simulink model "MCVT_Integration"  (R2020a)
settings: Solver=ode8  StartTime=0.0  StopTime=T_end  FixedStep=dt  RelTol=1e-3  EnableMultiTasking=on
values from neighbouring files (symbols this model uses):
  assumed_power_voltage = 1.25e2    (= 125)    [MCVT_Input_File.m]    (also in MCVT_Run_QuickCheck.m)
  battery_power_toggle = 1    [MCVT_Input_File.m]    (also in MCVT_Run_QuickCheck.m)
  battery_trickle_charge_param = 0.1    [MCVT_Input_File.m]    (also in MCVT_Run_QuickCheck.m)
  battery_trickle_leakage_param = 0.01    [MCVT_Input_File.m]    (also in MCVT_Run_QuickCheck.m)
  damage_case_id = 3    [MCVT_Input_File.m]    (also in MCVT_Run_QuickCheck.m = 1)
  dt = 1/fs    (= 0.001)    [MCVT_Run_QuickCheck.m]    (also in MCVT_Sim_Set.m)
  dt_2 = 1/fs_2    (= 0.001)    [MCVT_Run_QuickCheck.m]    (also in MCVT_Sim_Set.m)
  dt_3 = 1/fs_3    (= 0.001)    [MCVT_Run_QuickCheck.m]    (also in MCVT_Sim_Set.m)
  dt_5 = 1/fs_5    (= 0.005)    [MCVT_Run_QuickCheck.m]    (also in MCVT_Sim_Set.m)
  dt_8 = 1/fs_8    (= 0.05)    [MCVT_Run_QuickCheck.m]    (also in MCVT_Sim_Set.m)
  dt_9 = 1/fs_9    (= 0.001)    [MCVT_Run_QuickCheck.m]    (also in MCVT_Sim_Set.m)
  dust_met_duration = 3    [MCVT_Input_File.m]    (also in MCVT_Run_QuickCheck.m = 2)
  ECLSS_indoor_set_temperature = 298.15    [MCVT_Input_File.m]    (also in MCVT_Run_QuickCheck.m)
  energy_store_max_o = 150    [MCVT_Input_File.m]    (also in MCVT_Run_QuickCheck.m)
  energy_store_o = 120    [MCVT_Input_File.m]    (also in MCVT_Run_QuickCheck.m)
  fs = 1000    [MCVT_Run_QuickCheck.m]    (also in MCVT_Sim_Set.m)
  gain_charge_param = 1    [MCVT_Input_File.m]    (also in MCVT_Run_QuickCheck.m)
  ground_temperature = 373    [MCVT_Habitat_Design_Input_File.m]    (also in MCVT_Run_QuickCheck.m)
  I_max_charge = 800    [MCVT_Input_File.m]    (also in MCVT_Run_QuickCheck.m)
  I_max_discharge = 800    [MCVT_Input_File.m]    (also in MCVT_Run_QuickCheck.m)
  initial_pressure = 1e5    (= 100000)    [MCVT_Input_File.m]    (also in MCVT_Run_QuickCheck.m)
  initial_temperature = 295    [MCVT_Input_File.m]    (also in MCVT_Run_QuickCheck.m)
  interior_leak_rate = 0.00415    [MCVT_Input_File.m]    (also in MCVT_Run_QuickCheck.m)
  launch_dust_accum_start_time = 10    [MCVT_Input_File.m]    (also in MCVT_Run_QuickCheck.m = 2)
  launch_dust_accum_stop_time = 12    [MCVT_Input_File.m]    (also in MCVT_Run_QuickCheck.m = 3)
  meteorite_impact_time = 2    [MCVT_Input_File.m]    (also in MCVT_Run_QuickCheck.m = 1)
  nuclear_power_toggle = 1    [MCVT_Input_File.m]    (also in MCVT_Run_QuickCheck.m)
  print_interval = 30    [MCVT_Input_File.m]
  SOC_lower_thres = 0.5    [MCVT_Input_File.m]    (also in MCVT_Run_QuickCheck.m)
  SOC_upper_thres = 0.9    [MCVT_Input_File.m]    (also in MCVT_Run_QuickCheck.m)
  solar_power_toggle = 1    [MCVT_Input_File.m]    (also in MCVT_Run_QuickCheck.m)
  space_temperature = 4    [MCVT_Habitat_Design_Input_File.m]    (also in MCVT_Run_QuickCheck.m)
  Start_angle = 90    [MCVT_Input_File.m]    (also in MCVT_Run_QuickCheck.m)
undefined symbols (not defined in the file or in its folder): A_flow_restrictor, ACTIVITY_LOOKUP_TABLE, air, Aref, Bmap, Bmap2, Bmap3, comms, DiscIntNeverNeededParam, DmgSPL, dust_parameters, ECLSS_dust_damage_repair_time, ECLSS_heater_capacity, ECLSS_IPS_restrictor, ECLSS_IPS_setpointPressure, ECLSS_paint_damage_repair_time, ECLSS_radiatorLoop_discreteNumber, ECLSS_Vst, emptyActivity, expected_heat_add, FI, habitat, HIEM2SMM, HIEM2STM, HIEMDamageElements, impact_numbering, initial_SPLT_damage_index, input_air, input_Cond, input_CP, input_discretization, input_EV, input_Evap, input_radiatorLoop, K_ECLSS_dust_single, K_ECLSS_paint_single, K_Structure_damage_single, Kref, kW_to_W, meteoroidDustDep, mFRF, ndelays, nominalDustDep, noverlap, nuclear_toggle_gain, output_limits, panel_paint0, Ps0, reference_temperature, RepairTime_ECLSS_factor, Sensor_Location_SMM, SMM_T0, SMM_T1, SMM_T2, SMM_T3, SMM_T4, SMM_T4_0, SMM_T4_1, SMM_T4_2, solar_FDD_K ... (+73)
libraries: neural x350, simulink x39, xpcutilitieslib x7, xpcrtudplib x7, dspstat3 x1
(part 43 of 43)

== matlab function MCVT_Integration/Sys5 - ECLSS/Interior Pressure Control System (IPCS)/Restrictor ==
  data: Ps input; Afr input; ms output; Pint input; Vop input; ECLSS_IPS_restrictor parameter; air parameter
function ms = flow_restrictor(Ps, Afr, Pint, Vop,  ECLSS_IPS_restrictor, air)
%% Documentation
% Purpose: find mass flow rate of air supply
% Date Created: 20 June 2020
% Date Last Modified: 27 July 2021
% Modeler Name: Jaewon Park, CJ Pan (Purdue)
% Funding Acknowledgement: Funded by the NASA RETHi Project (80NSSC19K1076)

% Version Number: MCVT v1.4

% Subsystem Connections: recieves an input from subsystem 8 and sends an output to subsystem 8

%%% All inputs and outputs to this function are 1 dimensional %%%
% INPUTS %
%   - Ps: air storage tank pressure, unit: Pa, type: float
%   - Pint: interior environment pressure, unit: Pa, type: float
%   - Afr: effective cross-sectional area of flow restrictor, unit: m^2, type: float
%   - Vop: valve opening factor, used for damageability case (TO DO)
%   - ECLSS_IPS_restrictor: flow restrictor design parameters, type: struct (see below for dot indices)
%                           permeability: Permeability of the flow restrictor, unit: m^2, type: float
%                           length: Length of the flow restrictor, unit:m, type: float
%                           channel number: number of small channels in restrictor, unit: none, type: float
%                           cross area: Flow restrictor area (cross-section), unit: m^2, type: float
%   - air: air material property, type: struct (see below for dot indices)
%                           specific heat: Specific heat of air, unit: J/(kg.k), type: float
%                           density: density of air, unit: kg/m^3, type: float
%                           viscosity: dynamic viscosity of air, unit: Pa*s, type: float
    
% OUTPUTS %
%   - ms: mass flow rate of air supply, unit: kg/s, type: float

% Function Dependencies: No dependency

% No Data Depenencies

%% Calculate mass flow rate through a flow restrictor
ms=ECLSS_IPS_restrictor.channel_number * Vop * Afr * air.density *...
    ECLSS_IPS_restrictor.permeability / air.viscosity * (Ps-Pint) / ...
    ECLSS_IPS_restrictor.length;                                        % mass flow rate of supplied air


end

== matlab function MCVT_Integration/Sys5 - ECLSS/Active Thermal Control System (ATCS)/tempadjust ==
  data: x input; y output; Tset input; t input
function y= tempadjust(x, Tset, t)
%% Documentation
% Purpose: Filter out temperature readings that are not a number 
% Date Created: 20 June 2020
% Date Last Modified: 1 Sept 2021
% Modeler Name: Yuguang Fu(Purdue)
% Funding Acknowledgement: Funded by the NASA RETHi Project (80NSSC19K1076)

% Version Number: MCVT v1.4

% Subsystem Connections: receives input from subsystem 8

%%% All inputs and outputs to this function are 1 dimensional %%%
% INPUTS %
%   - x: temperature reading from interior habitat, unit: C, type: float
%   - Tset: setpoint temperature, unit: C, type: float
%   - t: current simulation time, unit: s, type: float
    
% OUTPUTS %
%   - y: filtered temperature, unit: C, type: float

% Function Dependencies: No dependency

% No Data Depenencies
%% Filtering
    if isnan(x) && t<=0.105 % if the temperature reading is not a number and it is early stage of the simulation  
        %fprintf('Interior ENV temperature as nan')
        y=Tset;             % assume the reading is the same as temperature setpoint 
    elseif isnan(x)
        y=Tset;             % assume the reading is the same as temperature setpoint 
    else                    
        y=x;                % otherwise, just read the value as it is 
    end

    
% This is happening because at each subsystem is run at different
% frequencies. Interior habitat has the lowest frequency. Therefore, it
% does not output any value during the beginning stage of the simulation. 
% This problem needs to be solved (not by ECLSS obvisouly). This is only for temporary fix. 
end

== matlab function MCVT_Integration/Sys2 - Structural System/Sys2_Structural_System/Structural Mechanical 2.1/Cat Acceleration ==
  data: Acc input; Dom input; Acc_Sen output; SMM_T4_0 parameter; Sensor_Location_SMM parameter
function Acc_Sen = Cat_acceleration(Acc,Dom, SMM_T4_0,Sensor_Location_SMM)

%% This block extract acceleration for the given sensor location


%% SL contains sensor locations
% Select the closest nodes to given sensor locations
% Safe: Can work with any input coordinate
    [SN]=Sensor_Locator(Sensor_Location_SMM,SMM_T4_0.Nodes_M(:,:,Dom));
    
%% Get the acceleration only to the Sensors    
    Acc_Sen=zeros(length(SN)*3,1);
    for i=1:length(SN)
        
        Acc_Sen(3*i-2:3*i,1)=Acc(3*SN(i)-2:3*SN(i),1);
        
    end


end


function[SN,b]=Sensor_Locator(SL,Nodes)
   
    S=size(Nodes);
    nnp1=S(1);

    S=size(SL);
    SN=zeros(S(1),1);
    for i=1:S(1)
        
        D=zeros(nnp1,1);
        for k=1:nnp1
            D(k)=norm([Nodes(k,2) Nodes(k,3),Nodes(k,4)]-[SL(i,1),SL(i,2),SL(i,3)]);
        end
        
        [a,b]=min(D);
        
        SN(i)=Nodes(b,1);
    end 
        
end

== matlab function MCVT_Integration/Sys2 - Structural System/Sys2_Structural_System/Structural Mechanical 2.1/Domain Selection ==
  data: ILxyz input; Dom output; T_Node output; SMM_T4_0 parameter; T_NodeI input; DomI input; t input
function [Dom,T_Node] = Domain_Selection(ILxyz,SMM_T4_0,T_NodeI,DomI,t)
%% This block sets the Domain based on Impact location
% Inputs: 
%       ILxyz: Impact coordinate 
%       SMM_T4_0.P_N_M: Set of permissible Impact nodes on SMM
%       SMM_T4_0.Nodes_M: Matrix containing Node set. SMM_T4_0.Nodes_M(:,:,1) represents Nodes when Domain 1 forms Kbb matrix
%       T_NodeI: Target node input
%       DomI: Domain no input
%       t: Time

% Outputs:
%       Dom: Domain no output
%       T_Node: Target node output

%% Set previous as new to avoid Domain selection computation at each step

   T_Node=T_NodeI;  % Output Target Node set to Input Target Node (Impact node)
   Dom=DomI;        % Output Domain set to Input Domain

%% Select and Fix the domain only at the beginning of simulation
if t==0

    % This function searh for the closest Node and element 
        % Safe: Can work with any Impact location!
        [~,b]=Impact_Locator(ILxyz,SMM_T4_0.Nodes_M(SMM_T4_0.P_N_M(:,:,1),:,1));

    % Set the domain
        Dom=b;

    % Set the target node
        T_Node=SMM_T4_0.P_N_M(1,b,b);

end
    
    

end

%% Find closest node number SN and node array location b
function[SN,b]=Impact_Locator(SL,Nodes)

    % Input given node: SL format: [Node 1, Node 2,... Node i,... Node end]

    S=size(Nodes);
    nnp1=S(1);

    % If given SL Coordinates are in wrong format, make it right by transposing
    S=size(SL);
    if S(2)>1
        SL1=SL';
    else
        SL1=SL;
    end
    
    % Get the distance vector for each node
        D=zeros(nnp1,1);
        for k=1:nnp1
            D(k)=norm([Nodes(k,2) Nodes(k,3),Nodes(k,4)]-[SL1(1,1),SL1(2,1),SL1(3,1)]);
        end

    % Find the minimum
        [a,b]=min(D);

    % Set the Node number      
        SN=Nodes(b,1);

end

== matlab function MCVT_Integration/Sys2 - Structural System/Sys2_Structural_System/Structural Thermal Model 2.2/STM Temperature Sensors/ Resistance Temperature Detector1/T vs R ==
  data: R output; T input
function R = fcn(T)

if T >= 0
    R = 100*(1+3.9083*10^(-3)*T-5.775*10^(-7)*T^2);
else
    R = 100*(1+3.9083*10^(-3)*T-5.775*10^(-7)*T^2-4.183*10^(-12)*(T-100)*T^3);
end

== matlab function MCVT_Integration/Sys2 - Structural System/Sys2_Structural_System/Structural Thermal Model 2.2/STM Temperature Sensors/ Resistance Temperature Detector2/Power Consumption ==
  data: Supply input; kWUsage3 output; Switch input
function kWUsage3 = Power_Consumption(Supply,Switch)

if Switch >= 1
    Voltage = 5.5; %Volts
    Current = 0.000001; %10 microAmperes
    
    kWUsage3 = (Voltage * Current)/1000;
    
    if kWUsage3 >= Supply
        kWUsage3 = Supply;
    end
    
else
    kWUsage3 = 0;
end
end

== matlab function MCVT_Integration/Sys2 - Structural System/Sys2_Structural_System/Structural Thermal Model 2.2/STM Temperature Sensors/ Resistance Temperature Detector2/R vs T ==
  data: R input; T output
function T = fcn(R)

T = 1.76520388802152e-09 * R^4 - 2.98990866289637e-07 * R^3 + 0.000987249006463873 * R^2 ...
    + 2.36178487086887 * R - 245.924688605077;

end

== matlab function MCVT_Integration/Sys2 - Structural System/Sys2_Structural_System/Structural Thermal Model 2.2/STM Temperature Sensors/ Resistance Temperature Detector2/RTD with Self-heat Error/MATLAB Function ==
  data: I input; R input; U output
function U = fcn(I, R)

U = I * R;     % Operating current = 1mA
               % Voltage output U = I*R

== matlab function MCVT_Integration/Sys2 - Structural System/Sys2_Structural_System/Structural Thermal Model 2.2/STM Temperature Sensors/ Resistance Temperature Detector2/RTD without Self-heat Error/MATLAB Function ==
  data: I input; I output; R input; U output
function [I,U]= fcn(I,R)

U = I * R;     % Operating current = 1mA
               % Voltage output U = I*R

== matlab function MCVT_Integration/Sys2 - Structural System/Sys2_Structural_System/Structural Thermal Model 2.2/STM Temperature Sensors/ Resistance Temperature Detector2/Self-heat Calculation/  T vs R  ==
  data: R output; T input
function R = fcn(T)

if T >= 0
    R = 100*(1+3.9083*10^(-3)*T-5.775*10^(-7)*T^2);
else
    R = 100*(1+3.9083*10^(-3)*T-5.775*10^(-7)*T^2-4.183*10^(-12)*(T-100)*T^3);
end

== matlab function MCVT_Integration/Sys2 - Structural System/Sys2_Structural_System/Structural Thermal Model 2.2/STM Temperature Sensors/ Resistance Temperature Detector2/Switch ON//OFF Control Block/Switch OFF/MATLAB Function1 ==
  data: u input; y output; Time input
function y = fcn(u,Time)

if Time>=0
    u = NaN;
end

y = u;

== matlab function MCVT_Integration/Sys2 - Structural System/Sys2_Structural_System/Structural Thermal Model 2.2/STM Temperature Sensors/ Resistance Temperature Detector2/T vs R ==
  data: R output; T input
function R = fcn(T)

if T >= 0
    R = 100*(1+3.9083*10^(-3)*T-5.775*10^(-7)*T^2);
else
    R = 100*(1+3.9083*10^(-3)*T-5.775*10^(-7)*T^2-4.183*10^(-12)*(T-100)*T^3);
end

== matlab function MCVT_Integration/Sys2 - Structural System/Sys2_Structural_System/Structural Thermal Model 2.2/STM Temperature Sensors/ Switch ON//OFF Control Number4 ==
  data: Power input; Switch output
function Switch = Switch2(Power)

if Power <= 5.5e-9
    Switch = 2;
else
    Switch = 1;
end

end

== matlab function MCVT_Integration/Sys2 - Structural System/Sys2_Structural_System/Structural Thermal Model 2.2/STM Temperature Sensors/Cumulative_Power_STM ==
  data: TP input; CTP output; PrevCTP input
function CTP = Cumulative_Power_STM(TP,PrevCTP)

CTP = TP+PrevCTP;

end

== matlab function MCVT_Integration/Sys2 - Structural System/Sys2_Structural_System/Structural Thermal Model 2.2/STM Temperature Sensors/Sensor Input to CDCM ==
  data: RightBottom input; Wall_Temp_Sensed output; Zenith input; LeftBottom input
function Wall_Temp_Sensed = Sensor_Placement(RightBottom,Zenith,LeftBottom)

a = RightBottom(1,1);
b = Zenith(1,1);
c = LeftBottom(1,1);

Wall_Temp_Sensed = ones(3,1);

Wall_Temp_Sensed(1,1) = a;
Wall_Temp_Sensed(2,1) = b;
Wall_Temp_Sensed(3,1) = c;

end

== matlab function MCVT_Integration/Sys2 - Structural System/Sys2_Structural_System/Structural Thermal Model 2.2/STM Temperature Sensors/Sensor Placement ==
  data: Wall_Temp input; RightBottom output; Zenith output; LeftBottom output
function [RightBottom,Zenith,LeftBottom] = Sensor_Placement(Wall_Temp)

Locations = [1 5 11];

Temp_to_Sensor = Wall_Temp(Locations,1);

RightBottom = Temp_to_Sensor(1,1);
Zenith = Temp_to_Sensor(2,1);
LeftBottom = Temp_to_Sensor(3,1);

end

== matlab function MCVT_Integration/Sys 6 - Health Management and Agents/Decision Maker/Simulink Communication Modules/ECLSS_Dust//MCVT API to HMS/UDP_Send/MATLAB Function1 ==
  data: SizeInfo output; signal input
function SizeInfo=size_Info(signal)

info=size(signal);

SizeInfo=[info(1), info(2)];

== matlab function MCVT_Integration/Sys 6 - Health Management and Agents/Decision Maker/Simulink Communication Modules/ECLSS_Dust//MCVT API to HMS/module2 ==
  data: u_t input; u_av output; u_std output; buffSize input
function [u_av, u_std] = buffer_average(u_t, buffSize)

    if buffSize < 10
        buffSize = 10;
    end
    % initialize buffer
    [nR, ~] = size(u_t);
    
    persistent count;
    persistent u_arr;
    
    if isempty(count)
        count = 0;
        u_arr = zeros(buffSize,nR);
    end
    
    % shuffle the elements down
    u_arr(2:end,1:end) = u_arr(1:end-1,1:end);
    
    % add the new element
    u_arr(1,1:end) = u_t;
    
    % average
    if count == 0
        u_av = u_t;
        u_std = zeros(size(u_t));
        count = count + 1;
    elseif count < buffSize
        u_av = mean(u_arr(1:count+1, 1:end), 1)';
        u_std = std(u_arr(1:count+1, 1:end), 0, 1)';
        count = count + 1;
    else
        u_av = mean(u_arr, 1)';
        u_std = std(u_arr, 0, 1)';
        count = count + 1;
    end
        
end

== matlab function MCVT_Integration/Sys 6 - Health Management and Agents/Decision Maker/Simulink Communication Modules/ECLSS_Paint//MCVT API to HMS/UDP_Send/MATLAB Function ==
  data: hi0 output; len input; hdr_Info input; rowcol input; len0 output
function [hi0, len0]=headerInfo(len, hdr_Info, rowcol)
src=hdr_Info(1);
dest=hdr_Info(2);
type=hdr_Info(3);
prio=hdr_Info(4);
row=rowcol(1); 
col=rowcol(2);
% len=0; 
hi0=[src, dest, type, prio, row, col];
len0=len;
% hi=uint8(hi);

== matlab function MCVT_Integration/Sys 6 - Health Management and Agents/Decision Maker/Simulink Communication Modules/ECLSS_Paint//MCVT API to HMS/UDP_Send/MATLAB Function1 ==
  data: SizeInfo output; signal input
function SizeInfo=size_Info(signal)

info=size(signal);

SizeInfo=[info(1), info(2)];

== matlab function MCVT_Integration/Sys 6 - Health Management and Agents/Decision Maker/Simulink Communication Modules/ECLSS_Paint//MCVT API to HMS/module2 ==
  data: u_t input; u_av output; u_std output; buffSize input
function [u_av, u_std] = buffer_average(u_t, buffSize)

    if buffSize < 10
        buffSize = 10;
    end
    % initialize buffer
    [nR, ~] = size(u_t);
    
    persistent count;
    persistent u_arr;
    
    if isempty(count)
        count = 0;
        u_arr = zeros(buffSize,nR);
    end
    
    % shuffle the elements down
    u_arr(2:end,1:end) = u_arr(1:end-1,1:end);
    
    % add the new element
    u_arr(1,1:end) = u_t;
    
    % average
    if count == 0
        u_av = u_t;
        u_std = zeros(size(u_t));
        count = count + 1;
    elseif count < buffSize
        u_av = mean(u_arr(1:count+1, 1:end), 1)';
        u_std = std(u_arr(1:count+1, 1:end), 0, 1)';
        count = count + 1;
    else
        u_av = mean(u_arr, 1)';
        u_std = std(u_arr, 0, 1)';
        count = count + 1;
    end
        
end

== matlab function MCVT_Integration/Sys 6 - Health Management and Agents/Decision Maker/Simulink Communication Modules/NPG_Dust//MCVT API to HMS/Buffer Average ==
  data: u_t input; u_av output; u_std output; buffSize input
function [u_av, u_std] = buffer_average(u_t, buffSize)
    

    if buffSize < 10
        buffSize = 10;
    end
    % initialize buffer
    [nR, ~] = size(u_t);
    
    persistent count;
    persistent u_arr;
    
    if isempty(count)
        count = 0;
        u_arr = zeros(buffSize,nR);
    end
    
    % shuffle the elements down
    u_arr(2:end,1:end) = u_arr(1:end-1,1:end);
    
    % add the new element
    u_arr(1,1:end) = u_t;
    
    % average
    if count == 0
        u_av = u_t;
        u_std = zeros(size(u_t));
        count = count + 1;
    elseif count < buffSize
        u_av = mean(u_arr(1:count+1, 1:end), 1)';
        u_std = std(u_arr(1:count+1, 1:end), 0, 1)';
        count = count + 1;
    else
        u_av = mean(u_arr, 1)';
        u_std = std(u_arr, 0, 1)';
        count = count + 1;
    end
        
end

== matlab function MCVT_Integration/Sys2 - Structural System/Sys2_Structural_System/Structural Mechanical 2.1/Solver ==
  data: Disp1 output; Disp0 input; Acc0 input; Vel0 input; Kbar input; D_Eq input; Vel1 output; Acc1 output; KbarO output; D_EqO output; Ext_F input; In_Acc input; Fadd input; Repair_Status input; FaddO output; Damage_Status input; Damage_Status_O output; Dom input; Im_F input; t input; Total_Damage output; SMM_T0 parameter; SMM_T1 parameter; SMM_T4_1 parameter; SMM_T2 parameter; SMM_T3 parameter; SMM_T4 parameter
function [Disp1,Vel1,Acc1,KbarO,D_EqO,FaddO,Damage_Status_O,Total_Damage]=Solver_Scheme_Sim(Disp0,Acc0,Vel0,Kbar,D_Eq,Ext_F,In_Acc,Fadd,Repair_Status, Damage_Status, Dom,Im_F,t,SMM_T0, SMM_T1, SMM_T4_1, SMM_T2, SMM_T3, SMM_T4)


%% This block Solves 3D Structural dynamics Equation: Md^2u/dt^2+Cdu/dt+Ku=F and calculate Damage and Repair
% Inputs ( not from Main\Workspace):
%       Ext_F: External Force vector
%       In_Acc: External acceleration
%       Im_F: Impact force
%       Fadd: Additional Force vector to stabilize excitation during repair at previous timestep
%       Repair_Status: Repair data from Agent model
%       Dom: Domain no
%       t: Time
%       Kbar: Mass normalized Stiffness matrix at previous timestep
%       D_Eq: Damage matrix at previous timestep
%       Damage_Status: List format of Damage matrix
%       Disp0: Displacement at previous timestep
%       Vel0: Velocity at previous timestep
%       Acc0: Acceleration at previous timestep

% Inputs from Main\Workspace
%       SMM_T0, SMM_T1, SMM_T4_1, SMM_T2, SMM_T3, SMM_T4: Read SysFiles\Subs2\SMM_Readme.txt

% Outputs: 
%       Disp1: Displacement at current timestep
%       Vel1: Velocity at current timestep
%       Acc1: Acceleration at current timestep
%       FaddO: Additional Force vector to stabilize excitation during repair at current timestep
%       KbarO: Mass normalized Stiffness matrix at current timestep
%       D_EqO: Damage matrix at current timestep
%       Damage_StatusO: List format of Damage matrix at current timestep



%% Damp external force at the beginning of simulation
    % Purpose:
        % At t=0 there is no force. At t=dt, Thermal+Internal Pressure+Gravity acts on the model
        % This sudden force cause unwanted excitation to the model.
        % This section multiply a corrective term CorrM to damp the force where force gradually increases for 1 sec instead of dt sec.
    
    if t<=1 %1 sec
        % Fourth order damping fit constants
            p1 =       1.182;
            p2 =        -2.2;
            p3 =     -0.1348;
            p4 =       2.153;
            p5 =   3.385e-16;
        % Corrective term to multiply with external force
            CorrM= p1*t^4+p2*t^3+p3*t^2+p4*t +p5;

        % Set maximum multiplyer to 1
            if CorrM>1
               CorrM=1;
            end
        
        % Set the damped external force vector
            Ext_F=Ext_F*CorrM;   
    end


%% Equivalent nodal force from Acceleration calculation
    % Summary:
        % Force=Mass*Acceleration. 
        % SMM_T4.M_M(:,:,Dom): Mass matrix associated to Domain Dom
        % In_Acc(1:SMM_T3.L,1): Chop the acceleration to L dergrees of freedom
    
    ext_A=SMM_T4.M_M(:,:,Dom)*In_Acc(1:SMM_T3.L,1);
                        
%% The Newmark method (constant acceleration)    
    % Purpose:
        % This section solves Md^2u/dt^2+Cdu/dt+Ku=F 
        % For details look into the Documentation
        % Equivalent terms for this code
            % M             : SMM_T4.M_M(:,:,Dom)
            % C             : SMM_T4.C_M(:,:,Dom)
            % K             : SMM_T4.K_M(:,:,Dom)
            % Kbar          : Kbar(:,:,Dom)
            % (t+delt)^F    : Ext_F(1:SMM_T3.L,1)+ext_A
            % a0-a7         : SMM_T1.a0-SMM_T1.a7
            

    % Equivalent Force vector calculation
        Rbar=SMM_T4.M_M(:,:,Dom)*(SMM_T1.a0*Disp0+SMM_T1.a2*Vel0+SMM_T1.a3*Acc0)+SMM_T4.C_M(:,:,Dom)*(SMM_T1.a1*Disp0+SMM_T1.a4*Vel0+SMM_T1.a5*Acc0)+Fadd(1:SMM_T3.L,1)+(Ext_F(1:SMM_T3.L,1)+ext_A);  
          
        fi=Rbar(1:SMM_T4.Li,1);
        fb=Rbar(SMM_T4.Li+1:end);

    % Calculation for domain decomposition
        Kbb=Kbar(SMM_T4.Li+1:end,SMM_T4.Li+1:end,Dom);
        Rb=chol(Kbb-SMM_T4.Kbbr_M(:,:,Dom));
        f_eq_b=fb-SMM_T4.fbr_M(:,:,Dom)*fi;
        
    % Displacement vectors for each domain 
        db = Rb\(Rb'\f_eq_b);                                       
        di = SMM_T4.IKii_M(:,:,Dom)*(fi-SMM_T4.Kib_M(:,:,Dom)*db);

    % Total displacement vector
        Disp1=[di;db];    

    % Solve for the corresponding velocities and accelerations
        Acc1=SMM_T1.a0*(Disp1-Disp0)-SMM_T1.a2*Vel0-SMM_T1.a3*Acc0;
        Vel1=Vel0+SMM_T1.a7*Acc1+SMM_T1.a6*Acc0; 
        
            
%% Damage Calculation 
    % Purpose:
        % This section reduce stiffness from the impacted element to replicate Damage
        
        
    % Get the element that is impacted
        e=SMM_T0.Reg_Elems(Dom);   

    % ---------------Get damage from data driven model------------
    % Hard coded for efficiency
    % This data driven model has been developed around March 2021

    % Damage constant
        Im_F=abs(Im_F)/10^6;            % Impact force in MPA
        dam_26=48.52*Im_F^0.1331-40;    % Damage constant near outer surface
        dam_27=48.52*Im_F^0.1331-40-8;  % Damage constant at the middle of element
        dam_21=48.52*Im_F^0.1331-40-10; % Damage constant near inner surface

    % Damage volume (m^3)
        Vl=zeros(3,1);
        if dam_26>0
            Vl(1)=dam_26*0.133333^3*10; % Damage volume near outer surface
        end
        if dam_27>0
            Vl(2)=dam_27*0.133333^3*6;  % Damage volume at the middle of element
        end
        if dam_21>0
            Vl(3)=dam_21*0.133333^3*4.5;% Damage volume near inner surface
        end

    %------------Remove Stiffness from Element--------- 
    % Safe: Work with any Input model. Doesn't crash
    c=1;
    for gp=[26 27 21]   % 26, 27 and 21 Gauss points are at the center of element

        if sum(Vl)>0  && D_Eq(gp,e)==0% Switch calculation if Volume loss detected

            % Get total stiffness for the element e
                KeT=SMM_T3.Ke(1:SMM_T0.Type(2,e)*3,1:SMM_T0.Type(2,e)*3,gp,e);

            % Determine the Damage (Stiffness loss ratio) from Volume loss    
                Mag=(Vl(c)/(SMM_T2.dJB(gp,e)*SMM_T1.W(gp,SMM_T0.Type(1,e))));

            % Stop damage from exceeding 1.5
                if Mag>1.5
                    Mag=1.5;
                    fprintf('        Warning! Damage is too high! Restricted to 1.5\n');
                end

            % Updated stiffness matrix    
                KeU=KeT*(1-Mag);

            % Remove Gauss point Stiffness from Global stiffness
                for loop1 = 1:SMM_T0.Type(2,e)*3
                    k = SMM_T4_1.LM_M(loop1,e,Dom);
                    for loop2 =  1:SMM_T0.Type(2,e)*3
                       j = SMM_T4_1.LM_M(loop2,e,Dom);
                       if k<=SMM_T3.L && j<=SMM_T3.L % Exclude D.O.F. associated with fixed end Boundary
                           % New Kbar    = Previous Kbar+Updated K       -Total K    
                           Kbar(k,j,Dom) = Kbar(k,j,Dom)+KeU(loop1,loop2)-KeT(loop1,loop2);     
                        end
                    end
                end

            % Update damage to D_Eq
                D_Eq(gp,e)=Mag;

        end 
        c=c+1; % Keep track of V1 vector
    end            
            
            
%% Repair Calculation    
    % Purpose:
        % This section Add stiffness to the damaged element to replicate Repair
        % This also determines additional force vector Fadd to exclude excitation to to repair
        
    % Set repair rate
        Repair_Rate= Repair_Status(4,1);    % Get repair rate (m^3)/0.001sec

    % Distribute repair rate to damaged Gauss points
        S=length(find(D_Eq>10^-3));
        if S>0
            RateI=Repair_Rate/S; % Distribute repair amount among Damaged gauss points
        else
            RateI=Repair_Rate;   % When no Damaged gauss points are there the code still runs w/o error
        end

    %------------------Add Stiffness to Element due to repair--------- 
    % Safe: Work with any Input model. Repair never goes over healthy state.   
        if Repair_Status(1,1)>10^-3 % Switch on repair action when Agent is enganged. Repair_Status(1,1)=1 

            for gp=1:SMM_T0.Type(3,e)   % Runs through all the Gauss points

                if D_Eq(gp,e)>10^-5  % Switch on repair action further if Damage amount >Tolerance

                    % Determine the Repair (Stiffness Gain ratio) from Volume gain
                        Mag_rep=RateI/(SMM_T2.dJB(gp,e)*SMM_T1.W(gp,SMM_T0.Type(1,e))); 

                    % If Repaired amount exceeds healty amount adjust Repair 
                        RP=0;
                        if Mag_rep>D_Eq(gp,e)
                            Mag_rep=D_Eq(gp,e); % Set repair to make Damage=0
                            RP=1;
                        end

                    % Previous 1-Damage
                        MagP=1-D_Eq(gp,e);

                    % New 1-Damage
                        MagN=1-(D_Eq(gp,e)-Mag_rep);

                        if MagN>1
                            MagN=1;
                        end
                        
                    % Get total stiffness for the element e
                        KeT=SMM_T3.Ke(1:SMM_T0.Type(2,e)*3,1:SMM_T0.Type(2,e)*3,gp,e);

                    % Get Stiffness to be added to Kbar
                        dKe=(MagN-MagP)*KeT;

                    % Add Gauss point Stiffness to Global stiffness 
                        for loop1 = 1:SMM_T0.Type(2,e)*3
                            k = SMM_T4_1.LM_M(loop1,e,Dom);
                            for loop2 =  1:SMM_T0.Type(2,e)*3
                               j = SMM_T4_1.LM_M(loop2,e,Dom);
                               if k<=SMM_T3.L && j<=SMM_T3.L % Exclude Boundaru D.O.F.
                                   % New Kbar    = Old Kbar     +Added Kbar
                                   Kbar(k,j,Dom) = Kbar(k,j,Dom)+dKe(loop1,loop2);
                                end
                            end
                        end

                    % Set the Damage matrix D_Eq
                        if RP==1
                            D_Eq(gp,e)=0;
                        else
                            D_Eq(gp,e)=(D_Eq(gp,e)-Mag_rep);
                        end

                end
            end

            % Add Correction Force vector to damp acceleration 
                Fadd(1:SMM_T3.L)=Fadd(1:SMM_T3.L)+Kbar(:,:,Dom)*Disp1-Rbar; 


        end


%% Set damage output for other models
    % Purpose:
        % Convert Damage matrix D_Eq (Which is required for SMM computation) to a Vector: Damage_Status, which can be used by other subsystems
        
    i=1;
    % SM.Damage_Status=SM.Damage_Status*0;
    for e=1:SMM_T1.nel%1:SM.SMM_T1.nel%[1 2 9 10 17 18]%1:SM.SMM_T1.nel % CPU parallelization may be done, making multiple domains of elements                      
           for gp=1:SMM_T0.Type(3,e)  % Complete parallelization may be done with GPU, each GPU processor for each gauss point, the for loop for element may go away

                  Damage_Status(i,1)=[D_Eq(gp,e)];

               i=i+1;
           end
    end
               
                    
    Damage_Status_O=Damage_Status;
    Total_Damage=sum(sum(D_Eq));%sum(Damage_Status);
            
%% Setting output different name then input

    KbarO=Kbar;
    D_EqO=D_Eq;
    FaddO=Fadd;
            
end

== matlab function MCVT_Integration/Sys2 - Structural System/Sys2_Structural_System/Fault Detection and Diagnosis (FDD) with Binary Classifer/Accelerometer/Accelerometer/ Switch ON//OFF Control Number ==
  data: p input; y output
function y = fcn(p)

if p >= 1.2*10^(-4)*3
    u = 1;
else
    u = 2;
end

y = u;

== matlab function MCVT_Integration/Sys2 - Structural System/Sys2_Structural_System/Fault Detection and Diagnosis (FDD) with Binary Classifer/Accelerometer/Accelerometer/Accelerometer/Maximum//minimum Output Voltage ==
  data: u input; y output
function y = fcn(u)

y = u(1:9);

if u(1:9) > 17
    y = 17*ones(9,1);
else
    if u(1:9) < 7
        y = 7*ones(9,1);
    end
end

== matlab function MCVT_Integration/Sys2 - Structural System/Sys2_Structural_System/Fault Detection and Diagnosis (FDD) with Binary Classifer/Accelerometer/Accelerometer/Damage State Control Block ==
  data: state_number input; y output; Acc input; Time input
function y = fcn(state_number,Acc,Time)

for i = 1:size(state_number,1)
    if state_number(i) == 1
        Acc(i*3-2:i*3) = Acc(i*3-2:i*3);
    else
        if state_number(i) == 2
            temp = Acc(i*3-2:i*3) - 12;
            Acc(i*3-2:i*3) = temp * 0.1 + 12;
        else
            if state_number(i) == 3
                if Time>=11 && Time<=14
                    Acc(i*3-2:i*3) = NaN;
                end
            else
                if state_number(i) == 4
                    Acc(i*3-2:i*3) = NaN;
                end
            end
        end
    end
end

y = Acc;

== matlab function MCVT_Integration/Sys2 - Structural System/Sys2_Structural_System/Fault Detection and Diagnosis (FDD) with Binary Classifer/Accelerometer/Accelerometer/Switch ON//OFF Control Block/Switch OFF/MATLAB Function1 ==
  data: sensor input; u input; y output; Time input
function y = fcn(sensor,u,Time)

for i = 1:size(sensor,1)
    if Time>=0
        u(sensor(i)*3-2:sensor(i)*3) = NaN;
    end
end

y = u;

== matlab function MCVT_Integration/Sys2 - Structural System/Sys2_Structural_System/Fault Detection and Diagnosis (FDD) with Binary Classifer/Accelerometer Power Consumption ==
  data: p input; y output
function y = fcn(p)

if p >= 1.2*10^(-4)*3
    u = 1.2*10^(-4)*3;
else
    u = 0;
end

y = u;

== matlab function MCVT_Integration/Sys2 - Structural System/Sys2_Structural_System/Fault Detection and Diagnosis (FDD) with Binary Classifer/Data Anomaly Detection/Data Anomaly Detection ==
  data: Acc input; indicator output; max_value output; num_nan output; y output
function [indicator,max_value,num_nan,y] = fcn(Acc)

indicator = zeros(9,1);
num_nan = zeros(9,1);
max_value = zeros(9,1);

for i = 1:9
    
    y = Acc(:,i);
    TF = isnan(Acc(:,i));
    num_nan(i) = sum(TF);
    max_value(i) = max(abs(Acc(:,i)));
    
    if num_nan(i) == 0
        indicator(i) = 1;
    else
        if num_nan(i) >= 1 && num_nan(i) < 5120
            indicator(i) = 3;
        else
            if num_nan(i) == 5120
                indicator(i) = 4;
            end
        end
    end
    
    if indicator(i) == 1 && max_value(i) <= 3e-3 || max_value(i) >= 1   % modified to 1
        indicator(i) = 2;
    end   
end

== matlab function MCVT_Integration/Sys2 - Structural System/Sys2_Structural_System/Fault Detection and Diagnosis (FDD) with Binary Classifer/FRF from Accelerometer 1/FRF Calculation ==
  data: exc input; frf output; acc input; m input; noverlap input; w input
function frf = xfunc(exc,acc,m,noverlap,w)

%  [XFER21,FREQV,P] = XFUNC(X,Y,FS,M,NOVERLAP) will estimate the
%	complex transfer function given time histories of the
%	input, X, and the output, Y. Given the complex response,
%	the magnitude and phase can be determined by using the
%	ABS and ANGLE functions. FS is the sampling frequency (Hz)
%	at which the data was taken. The X and Y sequences of
%	N points are divided into NS sections of M points each
%	(M must be a power of two). Using an M-point FFT,
%	successive sections are Hanning windowed, FFT'd and
%	averaged.	** SOME PARTS FROM SPECTRUM FUNCTION
%
%	XFUNC(X,Y,FS) 	--> NO AVERAGING
%	XFUNC(X,Y,FS,M)	--> AVERAGING BUT NO OVERLAP
%	NO OUTPUT ARGUMENTS WILL CREATE A BODE PLOT
%
% 	The output P is the matrix of power spectrums in the order
%	P = [Pxx,Pyy,Pxy].
%
%	NOTES: 	Xfer data may be written to a specified file.
%		Variables were chosen to immitate certain IP names.
%		Depending on the anti-aliasing filter used a certain
%			percentage of the data will include aliased
%			data (IP is 78% correct).

% COMMENTS
% nargin = 3 --> no overlap, no averaging
% nargin = 4 --> no overlap, averaging data
% nargin = 5 --> some overlap, and averaging data
% set color variable to easily change plot color
% may also write data to a file

frf = zeros(1,256*3);
x = exc;

for k = 1:1:3
    y = acc(:,k);
    
%     color	= 'b';
%     
%     if (nargin == 3), m = length(y); noverlap = 0; fmax = fs/2; end
%     if (nargin == 4)
%         if (length(y) == 1)
%             noverlap = m;
%             m = length(y);
%         else
%             noverlap = 0;
%         end
%         fmax = fs/2;
%     end
    %keyboard
    x 	= x(:);		% Make sure x and y are column vectors
    y 	= y(:);
    
    % DEFINE CONSTANTS
     n 	= max(size(x));	% Number of data points in entire time history
     ns 	= fix((n-noverlap)/(m-noverlap));	% Number of samples (frames)
    % (k = fix(n/m) for noverlap=0)
%     w 	= hann(m);	% hanning is replaced by hann
    % Type of windowing: change this for application
    % (Try HAMMING, BLACKMAN, BARTLETT, or your own)
    % HANNING is best for narrow-band random !!
     KMU 	= ns*norm(w)^2;	% Norm. scale factor (AVERAGING & WINDOWING)
     index 	= 1:m;
    % fprintf(1,['\n%5i',' Samples Averaged\n'],ns)
    
    
    % FIND POWER SPECTRUMS OF EACH SAMPLE AND SUM
    Pxx = zeros(m,1)+0i; 
%     Pyy = Pxx; 
    Pxy = Pxx; % Plus 0i
    for i = 1:ns
        xw 	= w.*x(index);		% detrend? no difference
        yw 	= w.*y(index);
        index 	= index + (m - noverlap);
        Xx 	= fft(xw);
        Yy 	= fft(yw);
        Pxx 	= Pxx + Xx.*conj(Xx);
%         Pyy 	= Pyy + Yy.*conj(Yy);
        Pxy 	= Pxy + Yy.*conj(Xx);
    end
    
    % SELECT
    %select = [1:m/2];
    select = [1:m/2+1];   % include DC AND Nyquist
    %FreqV	= [fs/2/(m/2):fs/2/(m/2):fs/2]';		% (Nyquist)
%     FreqV	= (select - 1)'*fs/m;
%     mcorrect	= round(.78*max(select));		% first 78%
%     fcorrect	= FreqV(mcorrect);
    
    % AVERAGED & SCALED DATA
    Pxx = Pxx(select)./KMU;			% Double sided
%     Pyy = Pyy(select)./KMU;
    Pxy = Pxy(select)./KMU;
%     P   = [Pxx,Pyy,Pxy];
    
    Xfer21 	= Pxy./Pxx;
    frf(1,256*(k-1)+1:256*k) = abs(Xfer21(1:256,1));
end

% %keyboard
% % NO OUTPUT ARGUMENTS, PLOT BODE
% if (nargout == 0),
%     
%     fig1	= gcf;
%     subplot(211)
%     plot(FreqV,db(Xfer21),color);grid
%     xlabel('Frequency (Hz)')
%     ylabel('Gain (dB)')
%     title('Transfer Function')
%     limits1	= axis;hold on
%     plot([fcorrect,fcorrect],[limits1(3),limits1(4)],'r');
%     xlim([0,fmax]);
%     
%     hold off
%     
%     subplot(212)
%     plot(FreqV,angle(Xfer21)*180/pi,color);grid
%     xlabel('Frequency (Hz)')
%     ylabel('Phase (deg)')
%     limits2	= axis;hold on
%     plot([fcorrect,fcorrect],[limits2(3),limits2(4)],'r');
%     hold off
%     axis([0 fmax -200 50]);
%     %	orient tall;print
%     return % Suppress output
% end

== matlab function MCVT_Integration/Sys2 - Structural System/Sys2_Structural_System/Fault Detection and Diagnosis (FDD) with Binary Classifer/FRF from Accelerometer 2/FRF Calculation ==
  data: exc input; frf output; acc input; m input; noverlap input; w input
function frf = xfunc(exc,acc,m,noverlap,w)

%  [XFER21,FREQV,P] = XFUNC(X,Y,FS,M,NOVERLAP) will estimate the
%	complex transfer function given time histories of the
%	input, X, and the output, Y. Given the complex response,
%	the magnitude and phase can be determined by using the
%	ABS and ANGLE functions. FS is the sampling frequency (Hz)
%	at which the data was taken. The X and Y sequences of
%	N points are divided into NS sections of M points each
%	(M must be a power of two). Using an M-point FFT,
%	successive sections are Hanning windowed, FFT'd and
%	averaged.	** SOME PARTS FROM SPECTRUM FUNCTION
%
%	XFUNC(X,Y,FS) 	--> NO AVERAGING
%	XFUNC(X,Y,FS,M)	--> AVERAGING BUT NO OVERLAP
%	NO OUTPUT ARGUMENTS WILL CREATE A BODE PLOT
%
% 	The output P is the matrix of power spectrums in the order
%	P = [Pxx,Pyy,Pxy].
%
%	NOTES: 	Xfer data may be written to a specified file.
%		Variables were chosen to immitate certain IP names.
%		Depending on the anti-aliasing filter used a certain
%			percentage of the data will include aliased
%			data (IP is 78% correct).

% COMMENTS
% nargin = 3 --> no overlap, no averaging
% nargin = 4 --> no overlap, averaging data
% nargin = 5 --> some overlap, and averaging data
% set color variable to easily change plot color
% may also write data to a file

frf = zeros(1,256*3);
x = exc;

for k = 1:1:3
    t = k+3;
    y = acc(:,t);
    
%     color	= 'b';
%     
%     if (nargin == 3), m = length(y); noverlap = 0; fmax = fs/2; end
%     if (nargin == 4)
%         if (length(y) == 1)
%             noverlap = m;
%             m = length(y);
%         else
%             noverlap = 0;
%         end
%         fmax = fs/2;
%     end
    %keyboard
    x 	= x(:);		% Make sure x and y are column vectors
    y 	= y(:);
    
    % DEFINE CONSTANTS
     n 	= max(size(x));	% Number of data points in entire time history
     ns 	= fix((n-noverlap)/(m-noverlap));	% Number of samples (frames)
    % (k = fix(n/m) for noverlap=0)
%     w 	= hann(m);	% hanning is replaced by hann
    % Type of windowing: change this for application
    % (Try HAMMING, BLACKMAN, BARTLETT, or your own)
    % HANNING is best for narrow-band random !!
     KMU 	= ns*norm(w)^2;	% Norm. scale factor (AVERAGING & WINDOWING)
     index 	= 1:m;
    % fprintf(1,['\n%5i',' Samples Averaged\n'],ns)
    
    
    % FIND POWER SPECTRUMS OF EACH SAMPLE AND SUM
    Pxx = zeros(m,1)+0i; 
%     Pyy = Pxx; 
    Pxy = Pxx; % Plus 0i
    for i = 1:ns
        xw 	= w.*x(index);		% detrend? no difference
        yw 	= w.*y(index);
        index 	= index + (m - noverlap);
        Xx 	= fft(xw);
        Yy 	= fft(yw);
        Pxx 	= Pxx + Xx.*conj(Xx);
%         Pyy 	= Pyy + Yy.*conj(Yy);
        Pxy 	= Pxy + Yy.*conj(Xx);
    end
    
    % SELECT
    %select = [1:m/2];
    select = [1:m/2+1];   % include DC AND Nyquist
    %FreqV	= [fs/2/(m/2):fs/2/(m/2):fs/2]';		% (Nyquist)
%     FreqV	= (select - 1)'*fs/m;
%     mcorrect	= round(.78*max(select));		% first 78%
%     fcorrect	= FreqV(mcorrect);
    
    % AVERAGED & SCALED DATA
    Pxx = Pxx(select)./KMU;			% Double sided
%     Pyy = Pyy(select)./KMU;
    Pxy = Pxy(select)./KMU;
%     P   = [Pxx,Pyy,Pxy];
    
    Xfer21 	= Pxy./Pxx;
    frf(1,256*(k-1)+1:256*k) = abs(Xfer21(1:256,1));
end

% %keyboard
% % NO OUTPUT ARGUMENTS, PLOT BODE
% if (nargout == 0),
%     
%     fig1	= gcf;
%     subplot(211)
%     plot(FreqV,db(Xfer21),color);grid
%     xlabel('Frequency (Hz)')
%     ylabel('Gain (dB)')
%     title('Transfer Function')
%     limits1	= axis;hold on
%     plot([fcorrect,fcorrect],[limits1(3),limits1(4)],'r');
%     xlim([0,fmax]);
%     
%     hold off
%     
%     subplot(212)
%     plot(FreqV,angle(Xfer21)*180/pi,color);grid
%     xlabel('Frequency (Hz)')
%     ylabel('Phase (deg)')
%     limits2	= axis;hold on
%     plot([fcorrect,fcorrect],[limits2(3),limits2(4)],'r');
%     hold off
%     axis([0 fmax -200 50]);
%     %	orient tall;print
%     return % Suppress output
% end

== matlab function MCVT_Integration/Sys 6 - Health Management and Agents/Decision Maker/Simulink Communication Modules/NPG_Dust//MCVT API to HMS/UDP_Send/MATLAB Function ==
  data: hi0 output; len input; hdr_Info input; rowcol input; len0 output
function [hi0, len0]=headerInfo(len, hdr_Info, rowcol)
src=hdr_Info(1);
dest=hdr_Info(2);
type=hdr_Info(3);
prio=hdr_Info(4);
row=rowcol(1); 
col=rowcol(2);
% len=0; 
hi0=[src, dest, type, prio, row, col];
len0=len;
% hi=uint8(hi);

== matlab function MCVT_Integration/Sys 6 - Health Management and Agents/Decision Maker/Simulink Communication Modules/NPG_Dust//MCVT API to HMS/UDP_Send/MATLAB Function1 ==
  data: SizeInfo output; signal input
function SizeInfo=size_Info(signal)

info=size(signal);

SizeInfo=[info(1), info(2)];

== matlab function MCVT_Integration/Sys 6 - Health Management and Agents/Decision Maker/Simulink Communication Modules/Solar_PV//MCVT API to HMS/Buffer Average ==
  data: u_t input; u_av output; u_std output; buffSize input
function [u_av, u_std] = buffer_average(u_t, buffSize)
    

    if buffSize < 10
        buffSize = 10;
    end
    % initialize buffer
    [nR, ~] = size(u_t);
    
    persistent count;
    persistent u_arr;
    
    if isempty(count)
        count = 0;
        u_arr = zeros(buffSize,nR);
    end
    
    % shuffle the elements down
    u_arr(2:end,1:end) = u_arr(1:end-1,1:end);
    
    % add the new element
    u_arr(1,1:end) = u_t;
    
    % average
    if count == 0
        u_av = u_t;
        u_std = zeros(size(u_t));
        count = count + 1;
    elseif count < buffSize
        u_av = mean(u_arr(1:count+1, 1:end), 1)';
        u_std = std(u_arr(1:count+1, 1:end), 0, 1)';
        count = count + 1;
    else
        u_av = mean(u_arr, 1)';
        u_std = std(u_arr, 0, 1)';
        count = count + 1;
    end
        
end

== matlab function MCVT_Integration/Sys 6 - Health Management and Agents/Decision Maker/Simulink Communication Modules/Solar_PV//MCVT API to HMS/UDP_Send/MATLAB Function ==
  data: hi0 output; len input; hdr_Info input; rowcol input; len0 output
function [hi0, len0]=headerInfo(len, hdr_Info, rowcol)
src=hdr_Info(1);
dest=hdr_Info(2);
type=hdr_Info(3);
prio=hdr_Info(4);
row=rowcol(1); 
col=rowcol(2);
% len=0; 
hi0=[src, dest, type, prio, row, col];
len0=len;
% hi=uint8(hi);

== matlab function MCVT_Integration/Sys 6 - Health Management and Agents/Decision Maker/Simulink Communication Modules/Solar_PV//MCVT API to HMS/UDP_Send/MATLAB Function1 ==
  data: SizeInfo output; signal input
function SizeInfo=size_Info(signal)

info=size(signal);

SizeInfo=[info(1), info(2)];

== matlab function MCVT_Integration/Sys 6 - Health Management and Agents/Decision Maker/Simulink Communication Modules/Structure_Dmg//MCVT API to HMS/Buffer Average ==
  data: u_t input; u_av output; u_std output; buffSize input
function [u_av, u_std] = buffer_average(u_t, buffSize)

    buffSize = max(10, buffSize);
    % initialize buffer
    [nR, ~] = size(u_t);
    
    persistent count;
    persistent u_arr;
    
    if isempty(count)
        count = 0;
        u_arr = zeros(buffSize,nR);
    end
    
    % shuffle the elements down
    u_arr(2:end,1:end) = u_arr(1:end-1,1:end);
    
    % add the new element
    u_arr(1,1:end) = u_t;
    
    % average
    if count == 0
        u_av = u_t;
        u_std = zeros(size(u_t));
        count = count + 1;
    elseif count < buffSize
        u_av = mean(u_arr(1:count+1, 1:end), 1)';
        u_std = std(u_arr(1:count+1, 1:end), 0, 1)';
        count = count + 1;
    else
        u_av = mean(u_arr, 1)';
        u_std = std(u_arr, 0, 1)';
        count = count + 1;
    end
        
end

== matlab function MCVT_Integration/Sys 6 - Health Management and Agents/Decision Maker/Simulink Communication Modules/Structure_Dmg//MCVT API to HMS/UDP_Send/MATLAB Function ==
  data: hi0 output; len input; hdr_Info input; rowcol input; len0 output
function [hi0, len0]=headerInfo(len, hdr_Info, rowcol)
src=hdr_Info(1);
dest=hdr_Info(2);
type=hdr_Info(3);
prio=hdr_Info(4);
row=rowcol(1); 
col=rowcol(2);
% len=0; 
hi0=[src, dest, type, prio, row, col];
len0=len;
% hi=uint8(hi);
